simulink model "complex_iq_bw2048_out32_fixaxi_v12b"  (R2019a) (continued)
== system complex_iq_bw2048_out32_fixaxi_v12b/dec_fir2 == (continued)
      |         set_param_state(gcb, 'frac_width', 'on')
      |     else
      |         set_param_state(gcb, 'exp_width', 'off')
      |         set_param_state(gcb, 'frac_width', 'off')
      |     end
      | 
      | 
      | else
      |     enable_fixpt = 'on'
      |     set_param_state(gcb, 'float_type', 'off')
      |     set_param_state(gcb, 'exp_width', 'off')
      |     set_param_state(gcb, 'frac_width', 'off')
      | end
      mask TypeOptions float_type: single | custom
      mask Callback float_type:
      | f_type = get_param(gcb, 'float_type')
      | if strcmp(f_type,'custom')
      |     set_param_state(gcb, 'exp_width', 'on')
      |     set_param_state(gcb, 'frac_width', 'on')
      | else
      |     set_param_state(gcb, 'exp_width', 'off')
      |     set_param_state(gcb, 'frac_width', 'off')
      | end
  ri_to_c : casper_library_misc/ri_to_c
      settings: SourceProductName=CASPER DSP Blockset
  shift1 : xbsIndex_r4/Shift  shift_dir=Left  shift_bits=1  en=off  latency=1  precision=Full  arith_type="Signed  (2's comp)"  n_bits=16  bin_pt=14  quantization=Truncate  overflow=Wrap  dbl_ovrd=off  block_type=shift
  shift2 : xbsIndex_r4/Shift  shift_dir=Left  shift_bits=1  en=off  latency=1  precision=Full  arith_type="Signed  (2's comp)"  n_bits=16  bin_pt=14  quantization=Truncate  overflow=Wrap  dbl_ovrd=off  block_type=shift
  sync_out : Outport
  dout : Outport  Port=2
  wires:
  real1/1 -> fir_dbl_col1/1(real1)
  imag1/1 -> fir_dbl_col1/2(imag1)
  real2/1 -> fir_dbl_col1/3(real2)
  imag2/1 -> fir_dbl_col1/4(imag2)
  real3/1 -> fir_dbl_col1/5(real3)
  imag3/1 -> fir_dbl_col1/6(imag3)
  real4/1 -> fir_dbl_col1/7(real4)
  imag4/1 -> fir_dbl_col1/8(imag4)
  real5/1 -> fir_dbl_col1/9(real5)
  imag5/1 -> fir_dbl_col1/10(imag5)
  real6/1 -> fir_dbl_col1/11(real6)
  imag6/1 -> fir_dbl_col1/12(imag6)
  real7/1 -> fir_dbl_col1/13(real7)
  imag7/1 -> fir_dbl_col1/14(imag7)
  real8/1 -> fir_dbl_col1/15(real8)
  imag8/1 -> fir_dbl_col1/16(imag8)
  fir_dbl_col1/33(real_sum) -> real_sum/2(din1)
  fir_dbl_col1/34(imag_sum) -> imag_sum/2(din1)
  fir_dbl_col1/1(real_out1) -> fir_dbl_col2/1(real1)
  fir_dbl_col1/2(imag_out1) -> fir_dbl_col2/2(imag1)
  fir_dbl_col1/3(real_out2) -> fir_dbl_col2/3(real2)
  fir_dbl_col1/4(imag_out2) -> fir_dbl_col2/4(imag2)
  fir_dbl_col1/5(real_out3) -> fir_dbl_col2/5(real3)
  fir_dbl_col1/6(imag_out3) -> fir_dbl_col2/6(imag3)
  fir_dbl_col1/7(real_out4) -> fir_dbl_col2/7(real4)
  fir_dbl_col1/8(imag_out4) -> fir_dbl_col2/8(imag4)
  fir_dbl_col1/9(real_out5) -> fir_dbl_col2/9(real5)
  fir_dbl_col1/10(imag_out5) -> fir_dbl_col2/10(imag5)
  fir_dbl_col1/11(real_out6) -> fir_dbl_col2/11(real6)
  fir_dbl_col1/12(imag_out6) -> fir_dbl_col2/12(imag6)
  fir_dbl_col1/13(real_out7) -> fir_dbl_col2/13(real7)
  fir_dbl_col1/14(imag_out7) -> fir_dbl_col2/14(imag7)
  fir_dbl_col1/15(real_out8) -> fir_dbl_col2/15(real8)
  fir_dbl_col1/16(imag_out8) -> fir_dbl_col2/16(imag8)
  fir_dbl_col2/33(real_sum) -> real_sum/3(din2)
  fir_dbl_col2/34(imag_sum) -> imag_sum/3(din2)
  fir_dbl_col2/1(real_out1) -> fir_dbl_col3/1(real1)
  fir_dbl_col2/2(imag_out1) -> fir_dbl_col3/2(imag1)
  fir_dbl_col2/3(real_out2) -> fir_dbl_col3/3(real2)
  fir_dbl_col2/4(imag_out2) -> fir_dbl_col3/4(imag2)
  fir_dbl_col2/5(real_out3) -> fir_dbl_col3/5(real3)
  fir_dbl_col2/6(imag_out3) -> fir_dbl_col3/6(imag3)
  fir_dbl_col2/7(real_out4) -> fir_dbl_col3/7(real4)
  fir_dbl_col2/8(imag_out4) -> fir_dbl_col3/8(imag4)
  fir_dbl_col2/9(real_out5) -> fir_dbl_col3/9(real5)
  fir_dbl_col2/10(imag_out5) -> fir_dbl_col3/10(imag5)
  fir_dbl_col2/11(real_out6) -> fir_dbl_col3/11(real6)
  fir_dbl_col2/12(imag_out6) -> fir_dbl_col3/12(imag6)
  fir_dbl_col2/13(real_out7) -> fir_dbl_col3/13(real7)
  fir_dbl_col2/14(imag_out7) -> fir_dbl_col3/14(imag7)
  fir_dbl_col2/15(real_out8) -> fir_dbl_col3/15(real8)
  fir_dbl_col2/16(imag_out8) -> fir_dbl_col3/16(imag8)
  fir_dbl_col3/33(real_sum) -> real_sum/4(din3)
  fir_dbl_col3/34(imag_sum) -> imag_sum/4(din3)
  fir_dbl_col3/1(real_out1) -> fir_dbl_col4/1(real1)
  fir_dbl_col3/2(imag_out1) -> fir_dbl_col4/2(imag1)
  fir_dbl_col3/3(real_out2) -> fir_dbl_col4/3(real2)
  fir_dbl_col3/4(imag_out2) -> fir_dbl_col4/4(imag2)
  fir_dbl_col3/5(real_out3) -> fir_dbl_col4/5(real3)
  fir_dbl_col3/6(imag_out3) -> fir_dbl_col4/6(imag3)
  fir_dbl_col3/7(real_out4) -> fir_dbl_col4/7(real4)
  fir_dbl_col3/8(imag_out4) -> fir_dbl_col4/8(imag4)
  fir_dbl_col3/9(real_out5) -> fir_dbl_col4/9(real5)
  fir_dbl_col3/10(imag_out5) -> fir_dbl_col4/10(imag5)
  fir_dbl_col3/11(real_out6) -> fir_dbl_col4/11(real6)
  fir_dbl_col3/12(imag_out6) -> fir_dbl_col4/12(imag6)
  fir_dbl_col3/13(real_out7) -> fir_dbl_col4/13(real7)
  fir_dbl_col3/14(imag_out7) -> fir_dbl_col4/14(imag7)
  fir_dbl_col3/15(real_out8) -> fir_dbl_col4/15(real8)
  fir_dbl_col3/16(imag_out8) -> fir_dbl_col4/16(imag8)
  fir_dbl_col4/33(real_sum) -> real_sum/5(din4)
  fir_dbl_col4/34(imag_sum) -> imag_sum/5(din4)
  fir_dbl_col4/1(real_out1) -> fir_dbl_col5/1(real1)
  fir_dbl_col4/2(imag_out1) -> fir_dbl_col5/2(imag1)
  fir_dbl_col4/3(real_out2) -> fir_dbl_col5/3(real2)
  fir_dbl_col4/4(imag_out2) -> fir_dbl_col5/4(imag2)
  fir_dbl_col4/5(real_out3) -> fir_dbl_col5/5(real3)
  fir_dbl_col4/6(imag_out3) -> fir_dbl_col5/6(imag3)
  fir_dbl_col4/7(real_out4) -> fir_dbl_col5/7(real4)
  fir_dbl_col4/8(imag_out4) -> fir_dbl_col5/8(imag4)
  fir_dbl_col4/9(real_out5) -> fir_dbl_col5/9(real5)
  fir_dbl_col4/10(imag_out5) -> fir_dbl_col5/10(imag5)
  fir_dbl_col4/11(real_out6) -> fir_dbl_col5/11(real6)
  fir_dbl_col4/12(imag_out6) -> fir_dbl_col5/12(imag6)
  fir_dbl_col4/13(real_out7) -> fir_dbl_col5/13(real7)
  fir_dbl_col4/14(imag_out7) -> fir_dbl_col5/14(imag7)
  fir_dbl_col4/15(real_out8) -> fir_dbl_col5/15(real8)
  fir_dbl_col4/16(imag_out8) -> fir_dbl_col5/16(imag8)
  fir_dbl_col5/33(real_sum) -> real_sum/6(din5)
  fir_dbl_col5/34(imag_sum) -> imag_sum/6(din5)
  fir_dbl_col5/1(real_out1) -> fir_dbl_col6/1(real1)
  fir_dbl_col5/2(imag_out1) -> fir_dbl_col6/2(imag1)
  fir_dbl_col5/3(real_out2) -> fir_dbl_col6/3(real2)
  fir_dbl_col5/4(imag_out2) -> fir_dbl_col6/4(imag2)
  fir_dbl_col5/5(real_out3) -> fir_dbl_col6/5(real3)
  fir_dbl_col5/6(imag_out3) -> fir_dbl_col6/6(imag3)
  fir_dbl_col5/7(real_out4) -> fir_dbl_col6/7(real4)
  fir_dbl_col5/8(imag_out4) -> fir_dbl_col6/8(imag4)
  fir_dbl_col5/9(real_out5) -> fir_dbl_col6/9(real5)
  fir_dbl_col5/10(imag_out5) -> fir_dbl_col6/10(imag5)
  fir_dbl_col5/11(real_out6) -> fir_dbl_col6/11(real6)
  fir_dbl_col5/12(imag_out6) -> fir_dbl_col6/12(imag6)
  fir_dbl_col5/13(real_out7) -> fir_dbl_col6/13(real7)
  fir_dbl_col5/14(imag_out7) -> fir_dbl_col6/14(imag7)
  fir_dbl_col5/15(real_out8) -> fir_dbl_col6/15(real8)
  fir_dbl_col5/16(imag_out8) -> fir_dbl_col6/16(imag8)
  fir_dbl_col6/33(real_sum) -> real_sum/7(din6)
  fir_dbl_col6/34(imag_sum) -> imag_sum/7(din6)
  fir_dbl_col6/1(real_out1) -> fir_dbl_col7/1(real1)
  fir_dbl_col6/2(imag_out1) -> fir_dbl_col7/2(imag1)
  fir_dbl_col6/3(real_out2) -> fir_dbl_col7/3(real2)
  fir_dbl_col6/4(imag_out2) -> fir_dbl_col7/4(imag2)
  fir_dbl_col6/5(real_out3) -> fir_dbl_col7/5(real3)
  fir_dbl_col6/6(imag_out3) -> fir_dbl_col7/6(imag3)
  fir_dbl_col6/7(real_out4) -> fir_dbl_col7/7(real4)
  fir_dbl_col6/8(imag_out4) -> fir_dbl_col7/8(imag4)
  fir_dbl_col6/9(real_out5) -> fir_dbl_col7/9(real5)
  fir_dbl_col6/10(imag_out5) -> fir_dbl_col7/10(imag5)
  fir_dbl_col6/11(real_out6) -> fir_dbl_col7/11(real6)
  fir_dbl_col6/12(imag_out6) -> fir_dbl_col7/12(imag6)
  fir_dbl_col6/13(real_out7) -> fir_dbl_col7/13(real7)
  fir_dbl_col6/14(imag_out7) -> fir_dbl_col7/14(imag7)
  fir_dbl_col6/15(real_out8) -> fir_dbl_col7/15(real8)
  fir_dbl_col6/16(imag_out8) -> fir_dbl_col7/16(imag8)
  fir_dbl_col7/33(real_sum) -> real_sum/8(din7)
  fir_dbl_col7/34(imag_sum) -> imag_sum/8(din7)
  fir_dbl_col7/1(real_out1) -> fir_dbl_col8/1(real1)
  fir_dbl_col7/2(imag_out1) -> fir_dbl_col8/2(imag1)
  fir_dbl_col7/3(real_out2) -> fir_dbl_col8/3(real2)
  fir_dbl_col7/4(imag_out2) -> fir_dbl_col8/4(imag2)
  fir_dbl_col7/5(real_out3) -> fir_dbl_col8/5(real3)
  fir_dbl_col7/6(imag_out3) -> fir_dbl_col8/6(imag3)
  fir_dbl_col7/7(real_out4) -> fir_dbl_col8/7(real4)
  fir_dbl_col7/8(imag_out4) -> fir_dbl_col8/8(imag4)
  fir_dbl_col7/9(real_out5) -> fir_dbl_col8/9(real5)
  fir_dbl_col7/10(imag_out5) -> fir_dbl_col8/10(imag5)
  fir_dbl_col7/11(real_out6) -> fir_dbl_col8/11(real6)
  fir_dbl_col7/12(imag_out6) -> fir_dbl_col8/12(imag6)
  fir_dbl_col7/13(real_out7) -> fir_dbl_col8/13(real7)
  fir_dbl_col7/14(imag_out7) -> fir_dbl_col8/14(imag7)
  fir_dbl_col7/15(real_out8) -> fir_dbl_col8/15(real8)
  fir_dbl_col7/16(imag_out8) -> fir_dbl_col8/16(imag8)
  fir_dbl_col8/33(real_sum) -> real_sum/9(din8)
  fir_dbl_col8/34(imag_sum) -> imag_sum/9(din8)
  fir_dbl_col8/1(real_out1) -> fir_dbl_col9/1(real1)
  fir_dbl_col8/2(imag_out1) -> fir_dbl_col9/2(imag1)
  fir_dbl_col8/3(real_out2) -> fir_dbl_col9/3(real2)
  fir_dbl_col8/4(imag_out2) -> fir_dbl_col9/4(imag2)
  fir_dbl_col8/5(real_out3) -> fir_dbl_col9/5(real3)
  fir_dbl_col8/6(imag_out3) -> fir_dbl_col9/6(imag3)
  fir_dbl_col8/7(real_out4) -> fir_dbl_col9/7(real4)
  fir_dbl_col8/8(imag_out4) -> fir_dbl_col9/8(imag4)
  fir_dbl_col8/9(real_out5) -> fir_dbl_col9/9(real5)
  fir_dbl_col8/10(imag_out5) -> fir_dbl_col9/10(imag5)
  fir_dbl_col8/11(real_out6) -> fir_dbl_col9/11(real6)
  fir_dbl_col8/12(imag_out6) -> fir_dbl_col9/12(imag6)
  fir_dbl_col8/13(real_out7) -> fir_dbl_col9/13(real7)
  fir_dbl_col8/14(imag_out7) -> fir_dbl_col9/14(imag7)
  fir_dbl_col8/15(real_out8) -> fir_dbl_col9/15(real8)
  fir_dbl_col8/16(imag_out8) -> fir_dbl_col9/16(imag8)
  fir_dbl_col9/33(real_sum) -> real_sum/10(din9)
  fir_dbl_col9/34(imag_sum) -> imag_sum/10(din9)
  fir_dbl_col9/1(real_out1) -> fir_dbl_col10/1(real1)
  fir_dbl_col9/2(imag_out1) -> fir_dbl_col10/2(imag1)
  fir_dbl_col9/3(real_out2) -> fir_dbl_col10/3(real2)
  fir_dbl_col9/4(imag_out2) -> fir_dbl_col10/4(imag2)
  fir_dbl_col9/5(real_out3) -> fir_dbl_col10/5(real3)
  fir_dbl_col9/6(imag_out3) -> fir_dbl_col10/6(imag3)
  fir_dbl_col9/7(real_out4) -> fir_dbl_col10/7(real4)
  fir_dbl_col9/8(imag_out4) -> fir_dbl_col10/8(imag4)
  fir_dbl_col9/9(real_out5) -> fir_dbl_col10/9(real5)
  fir_dbl_col9/10(imag_out5) -> fir_dbl_col10/10(imag5)
  fir_dbl_col9/11(real_out6) -> fir_dbl_col10/11(real6)
  fir_dbl_col9/12(imag_out6) -> fir_dbl_col10/12(imag6)
  fir_dbl_col9/13(real_out7) -> fir_dbl_col10/13(real7)
  fir_dbl_col9/14(imag_out7) -> fir_dbl_col10/14(imag7)
  fir_dbl_col9/15(real_out8) -> fir_dbl_col10/15(real8)
  fir_dbl_col9/16(imag_out8) -> fir_dbl_col10/16(imag8)
  fir_dbl_col10/33(real_sum) -> real_sum/11(din10)
  fir_dbl_col10/34(imag_sum) -> imag_sum/11(din10)
  fir_dbl_col10/1(real_out1) -> fir_dbl_col11/1(real1)
  fir_dbl_col10/2(imag_out1) -> fir_dbl_col11/2(imag1)
  fir_dbl_col10/3(real_out2) -> fir_dbl_col11/3(real2)
  fir_dbl_col10/4(imag_out2) -> fir_dbl_col11/4(imag2)
  fir_dbl_col10/5(real_out3) -> fir_dbl_col11/5(real3)
  fir_dbl_col10/6(imag_out3) -> fir_dbl_col11/6(imag3)
  fir_dbl_col10/7(real_out4) -> fir_dbl_col11/7(real4)
  fir_dbl_col10/8(imag_out4) -> fir_dbl_col11/8(imag4)
  fir_dbl_col10/9(real_out5) -> fir_dbl_col11/9(real5)
  fir_dbl_col10/10(imag_out5) -> fir_dbl_col11/10(imag5)
  fir_dbl_col10/11(real_out6) -> fir_dbl_col11/11(real6)
  fir_dbl_col10/12(imag_out6) -> fir_dbl_col11/12(imag6)
  fir_dbl_col10/13(real_out7) -> fir_dbl_col11/13(real7)
  fir_dbl_col10/14(imag_out7) -> fir_dbl_col11/14(imag7)
  fir_dbl_col10/15(real_out8) -> fir_dbl_col11/15(real8)
  fir_dbl_col10/16(imag_out8) -> fir_dbl_col11/16(imag8)
  fir_dbl_col11/33(real_sum) -> real_sum/12(din11)
  fir_dbl_col11/34(imag_sum) -> imag_sum/12(din11)
  fir_dbl_col11/1(real_out1) -> fir_dbl_col12/1(real1)
  fir_dbl_col11/2(imag_out1) -> fir_dbl_col12/2(imag1)
  fir_dbl_col11/3(real_out2) -> fir_dbl_col12/3(real2)
  fir_dbl_col11/4(imag_out2) -> fir_dbl_col12/4(imag2)
  fir_dbl_col11/5(real_out3) -> fir_dbl_col12/5(real3)
  fir_dbl_col11/6(imag_out3) -> fir_dbl_col12/6(imag3)
  fir_dbl_col11/7(real_out4) -> fir_dbl_col12/7(real4)
  fir_dbl_col11/8(imag_out4) -> fir_dbl_col12/8(imag4)
  fir_dbl_col11/9(real_out5) -> fir_dbl_col12/9(real5)
  fir_dbl_col11/10(imag_out5) -> fir_dbl_col12/10(imag5)
  fir_dbl_col11/11(real_out6) -> fir_dbl_col12/11(real6)
  fir_dbl_col11/12(imag_out6) -> fir_dbl_col12/12(imag6)
  fir_dbl_col11/13(real_out7) -> fir_dbl_col12/13(real7)
  fir_dbl_col11/14(imag_out7) -> fir_dbl_col12/14(imag7)
  fir_dbl_col11/15(real_out8) -> fir_dbl_col12/15(real8)
  fir_dbl_col11/16(imag_out8) -> fir_dbl_col12/16(imag8)
  fir_dbl_col12/33(real_sum) -> real_sum/13(din12)
  fir_dbl_col12/34(imag_sum) -> imag_sum/13(din12)
  fir_dbl_col12/1(real_out1) -> fir_dbl_col13/1(real1)
  fir_dbl_col12/2(imag_out1) -> fir_dbl_col13/2(imag1)
  fir_dbl_col12/3(real_out2) -> fir_dbl_col13/3(real2)
  fir_dbl_col12/4(imag_out2) -> fir_dbl_col13/4(imag2)
  fir_dbl_col12/5(real_out3) -> fir_dbl_col13/5(real3)
  fir_dbl_col12/6(imag_out3) -> fir_dbl_col13/6(imag3)
  fir_dbl_col12/7(real_out4) -> fir_dbl_col13/7(real4)
  fir_dbl_col12/8(imag_out4) -> fir_dbl_col13/8(imag4)
  fir_dbl_col12/9(real_out5) -> fir_dbl_col13/9(real5)
  fir_dbl_col12/10(imag_out5) -> fir_dbl_col13/10(imag5)
  fir_dbl_col12/11(real_out6) -> fir_dbl_col13/11(real6)
  fir_dbl_col12/12(imag_out6) -> fir_dbl_col13/12(imag6)
  fir_dbl_col12/13(real_out7) -> fir_dbl_col13/13(real7)
  fir_dbl_col12/14(imag_out7) -> fir_dbl_col13/14(imag7)
  fir_dbl_col12/15(real_out8) -> fir_dbl_col13/15(real8)
  fir_dbl_col12/16(imag_out8) -> fir_dbl_col13/16(imag8)
  fir_dbl_col13/33(real_sum) -> real_sum/14(din13)
  fir_dbl_col13/34(imag_sum) -> imag_sum/14(din13)
  fir_dbl_col13/1(real_out1) -> fir_dbl_col14/1(real1)
  fir_dbl_col13/2(imag_out1) -> fir_dbl_col14/2(imag1)
  fir_dbl_col13/3(real_out2) -> fir_dbl_col14/3(real2)
  fir_dbl_col13/4(imag_out2) -> fir_dbl_col14/4(imag2)
  fir_dbl_col13/5(real_out3) -> fir_dbl_col14/5(real3)
  fir_dbl_col13/6(imag_out3) -> fir_dbl_col14/6(imag3)
  fir_dbl_col13/7(real_out4) -> fir_dbl_col14/7(real4)
  fir_dbl_col13/8(imag_out4) -> fir_dbl_col14/8(imag4)
  fir_dbl_col13/9(real_out5) -> fir_dbl_col14/9(real5)
  fir_dbl_col13/10(imag_out5) -> fir_dbl_col14/10(imag5)
  fir_dbl_col13/11(real_out6) -> fir_dbl_col14/11(real6)
  fir_dbl_col13/12(imag_out6) -> fir_dbl_col14/12(imag6)
  fir_dbl_col13/13(real_out7) -> fir_dbl_col14/13(real7)
  fir_dbl_col13/14(imag_out7) -> fir_dbl_col14/14(imag7)
  fir_dbl_col13/15(real_out8) -> fir_dbl_col14/15(real8)
  fir_dbl_col13/16(imag_out8) -> fir_dbl_col14/16(imag8)
  fir_dbl_col14/33(real_sum) -> real_sum/15(din14)
  fir_dbl_col14/34(imag_sum) -> imag_sum/15(din14)
  fir_dbl_col14/1(real_out1) -> fir_dbl_col15/1(real1)
  fir_dbl_col14/2(imag_out1) -> fir_dbl_col15/2(imag1)
  fir_dbl_col14/3(real_out2) -> fir_dbl_col15/3(real2)
  fir_dbl_col14/4(imag_out2) -> fir_dbl_col15/4(imag2)
  fir_dbl_col14/5(real_out3) -> fir_dbl_col15/5(real3)
  fir_dbl_col14/6(imag_out3) -> fir_dbl_col15/6(imag3)
  fir_dbl_col14/7(real_out4) -> fir_dbl_col15/7(real4)
  fir_dbl_col14/8(imag_out4) -> fir_dbl_col15/8(imag4)
  fir_dbl_col14/9(real_out5) -> fir_dbl_col15/9(real5)
  fir_dbl_col14/10(imag_out5) -> fir_dbl_col15/10(imag5)
  fir_dbl_col14/11(real_out6) -> fir_dbl_col15/11(real6)
  fir_dbl_col14/12(imag_out6) -> fir_dbl_col15/12(imag6)
  fir_dbl_col14/13(real_out7) -> fir_dbl_col15/13(real7)
  fir_dbl_col14/14(imag_out7) -> fir_dbl_col15/14(imag7)
  fir_dbl_col14/15(real_out8) -> fir_dbl_col15/15(real8)
  fir_dbl_col14/16(imag_out8) -> fir_dbl_col15/16(imag8)
  fir_dbl_col15/33(real_sum) -> real_sum/16(din15)
  fir_dbl_col15/34(imag_sum) -> imag_sum/16(din15)
  fir_dbl_col15/1(real_out1) -> fir_dbl_col16/1(real1)
  fir_dbl_col15/2(imag_out1) -> fir_dbl_col16/2(imag1)
  fir_dbl_col15/3(real_out2) -> fir_dbl_col16/3(real2)
  fir_dbl_col15/4(imag_out2) -> fir_dbl_col16/4(imag2)
  fir_dbl_col15/5(real_out3) -> fir_dbl_col16/5(real3)
  fir_dbl_col15/6(imag_out3) -> fir_dbl_col16/6(imag3)
  fir_dbl_col15/7(real_out4) -> fir_dbl_col16/7(real4)
  fir_dbl_col15/8(imag_out4) -> fir_dbl_col16/8(imag4)
  fir_dbl_col15/9(real_out5) -> fir_dbl_col16/9(real5)
  fir_dbl_col15/10(imag_out5) -> fir_dbl_col16/10(imag5)
  fir_dbl_col15/11(real_out6) -> fir_dbl_col16/11(real6)
  fir_dbl_col15/12(imag_out6) -> fir_dbl_col16/12(imag6)
  fir_dbl_col15/13(real_out7) -> fir_dbl_col16/13(real7)
  fir_dbl_col15/14(imag_out7) -> fir_dbl_col16/14(imag7)
  fir_dbl_col15/15(real_out8) -> fir_dbl_col16/15(real8)
  fir_dbl_col15/16(imag_out8) -> fir_dbl_col16/16(imag8)
  fir_dbl_col16/33(real_sum) -> real_sum/17(din16)
  fir_dbl_col16/34(imag_sum) -> imag_sum/17(din16)
  fir_dbl_col16/1(real_out1) -> fir_dbl_col17/1(real1)
  fir_dbl_col16/2(imag_out1) -> fir_dbl_col17/2(imag1)
  fir_dbl_col16/3(real_out2) -> fir_dbl_col17/3(real2)
  fir_dbl_col16/4(imag_out2) -> fir_dbl_col17/4(imag2)
  fir_dbl_col16/5(real_out3) -> fir_dbl_col17/5(real3)
  fir_dbl_col16/6(imag_out3) -> fir_dbl_col17/6(imag3)
  fir_dbl_col16/7(real_out4) -> fir_dbl_col17/7(real4)
  fir_dbl_col16/8(imag_out4) -> fir_dbl_col17/8(imag4)
  fir_dbl_col16/9(real_out5) -> fir_dbl_col17/9(real5)
  fir_dbl_col16/10(imag_out5) -> fir_dbl_col17/10(imag5)
  fir_dbl_col16/11(real_out6) -> fir_dbl_col17/11(real6)
  fir_dbl_col16/12(imag_out6) -> fir_dbl_col17/12(imag6)
  fir_dbl_col16/13(real_out7) -> fir_dbl_col17/13(real7)
  fir_dbl_col16/14(imag_out7) -> fir_dbl_col17/14(imag7)
  fir_dbl_col16/15(real_out8) -> fir_dbl_col17/15(real8)
  fir_dbl_col16/16(imag_out8) -> fir_dbl_col17/16(imag8)
  fir_dbl_col17/33(real_sum) -> real_sum/18(din17)
  fir_dbl_col17/34(imag_sum) -> imag_sum/18(din17)
  fir_dbl_col17/1(real_out1) -> fir_dbl_col18/1(real1)
  fir_dbl_col17/2(imag_out1) -> fir_dbl_col18/2(imag1)
  fir_dbl_col17/3(real_out2) -> fir_dbl_col18/3(real2)
  fir_dbl_col17/4(imag_out2) -> fir_dbl_col18/4(imag2)
  fir_dbl_col17/5(real_out3) -> fir_dbl_col18/5(real3)
  fir_dbl_col17/6(imag_out3) -> fir_dbl_col18/6(imag3)
  fir_dbl_col17/7(real_out4) -> fir_dbl_col18/7(real4)
  fir_dbl_col17/8(imag_out4) -> fir_dbl_col18/8(imag4)
  fir_dbl_col17/9(real_out5) -> fir_dbl_col18/9(real5)
  fir_dbl_col17/10(imag_out5) -> fir_dbl_col18/10(imag5)
  fir_dbl_col17/11(real_out6) -> fir_dbl_col18/11(real6)
  fir_dbl_col17/12(imag_out6) -> fir_dbl_col18/12(imag6)
  fir_dbl_col17/13(real_out7) -> fir_dbl_col18/13(real7)
  fir_dbl_col17/14(imag_out7) -> fir_dbl_col18/14(imag7)
  fir_dbl_col17/15(real_out8) -> fir_dbl_col18/15(real8)
  fir_dbl_col17/16(imag_out8) -> fir_dbl_col18/16(imag8)
  fir_dbl_col18/33(real_sum) -> real_sum/19(din18)
  fir_dbl_col18/34(imag_sum) -> imag_sum/19(din18)
  fir_dbl_col18/1(real_out1) -> fir_dbl_col19/1(real1)
  fir_dbl_col18/2(imag_out1) -> fir_dbl_col19/2(imag1)
  fir_dbl_col18/3(real_out2) -> fir_dbl_col19/3(real2)
  fir_dbl_col18/4(imag_out2) -> fir_dbl_col19/4(imag2)
  fir_dbl_col18/5(real_out3) -> fir_dbl_col19/5(real3)
  fir_dbl_col18/6(imag_out3) -> fir_dbl_col19/6(imag3)
  fir_dbl_col18/7(real_out4) -> fir_dbl_col19/7(real4)
  fir_dbl_col18/8(imag_out4) -> fir_dbl_col19/8(imag4)
  fir_dbl_col18/9(real_out5) -> fir_dbl_col19/9(real5)
  fir_dbl_col18/10(imag_out5) -> fir_dbl_col19/10(imag5)
  fir_dbl_col18/11(real_out6) -> fir_dbl_col19/11(real6)
  fir_dbl_col18/12(imag_out6) -> fir_dbl_col19/12(imag6)
  fir_dbl_col18/13(real_out7) -> fir_dbl_col19/13(real7)
  fir_dbl_col18/14(imag_out7) -> fir_dbl_col19/14(imag7)
  fir_dbl_col18/15(real_out8) -> fir_dbl_col19/15(real8)
  fir_dbl_col18/16(imag_out8) -> fir_dbl_col19/16(imag8)
  fir_dbl_col19/33(real_sum) -> real_sum/20(din19)
  fir_dbl_col19/34(imag_sum) -> imag_sum/20(din19)
  fir_dbl_col19/1(real_out1) -> fir_dbl_col20/1(real1)
  fir_dbl_col19/2(imag_out1) -> fir_dbl_col20/2(imag1)
  fir_dbl_col19/3(real_out2) -> fir_dbl_col20/3(real2)
  fir_dbl_col19/4(imag_out2) -> fir_dbl_col20/4(imag2)
  fir_dbl_col19/5(real_out3) -> fir_dbl_col20/5(real3)
  fir_dbl_col19/6(imag_out3) -> fir_dbl_col20/6(imag3)
  fir_dbl_col19/7(real_out4) -> fir_dbl_col20/7(real4)
  fir_dbl_col19/8(imag_out4) -> fir_dbl_col20/8(imag4)
  fir_dbl_col19/9(real_out5) -> fir_dbl_col20/9(real5)
  fir_dbl_col19/10(imag_out5) -> fir_dbl_col20/10(imag5)
  fir_dbl_col19/11(real_out6) -> fir_dbl_col20/11(real6)
  fir_dbl_col19/12(imag_out6) -> fir_dbl_col20/12(imag6)
  fir_dbl_col19/13(real_out7) -> fir_dbl_col20/13(real7)
  fir_dbl_col19/14(imag_out7) -> fir_dbl_col20/14(imag7)
  fir_dbl_col19/15(real_out8) -> fir_dbl_col20/15(real8)
  fir_dbl_col19/16(imag_out8) -> fir_dbl_col20/16(imag8)
  fir_dbl_col20/33(real_sum) -> real_sum/21(din20)
  fir_dbl_col20/34(imag_sum) -> imag_sum/21(din20)
  fir_dbl_col20/1(real_out1) -> fir_dbl_col21/1(real1)
  fir_dbl_col20/2(imag_out1) -> fir_dbl_col21/2(imag1)
  fir_dbl_col20/3(real_out2) -> fir_dbl_col21/3(real2)
  fir_dbl_col20/4(imag_out2) -> fir_dbl_col21/4(imag2)
  fir_dbl_col20/5(real_out3) -> fir_dbl_col21/5(real3)
  fir_dbl_col20/6(imag_out3) -> fir_dbl_col21/6(imag3)
  fir_dbl_col20/7(real_out4) -> fir_dbl_col21/7(real4)
  fir_dbl_col20/8(imag_out4) -> fir_dbl_col21/8(imag4)
  fir_dbl_col20/9(real_out5) -> fir_dbl_col21/9(real5)
  fir_dbl_col20/10(imag_out5) -> fir_dbl_col21/10(imag5)
  fir_dbl_col20/11(real_out6) -> fir_dbl_col21/11(real6)
  fir_dbl_col20/12(imag_out6) -> fir_dbl_col21/12(imag6)
  fir_dbl_col20/13(real_out7) -> fir_dbl_col21/13(real7)
  fir_dbl_col20/14(imag_out7) -> fir_dbl_col21/14(imag7)
  fir_dbl_col20/15(real_out8) -> fir_dbl_col21/15(real8)
  fir_dbl_col20/16(imag_out8) -> fir_dbl_col21/16(imag8)
  fir_dbl_col21/33(real_sum) -> real_sum/22(din21)
  fir_dbl_col21/34(imag_sum) -> imag_sum/22(din21)
  fir_dbl_col21/1(real_out1) -> fir_dbl_col22/1(real1)
  fir_dbl_col21/2(imag_out1) -> fir_dbl_col22/2(imag1)
  fir_dbl_col21/3(real_out2) -> fir_dbl_col22/3(real2)
  fir_dbl_col21/4(imag_out2) -> fir_dbl_col22/4(imag2)
  fir_dbl_col21/5(real_out3) -> fir_dbl_col22/5(real3)
  fir_dbl_col21/6(imag_out3) -> fir_dbl_col22/6(imag3)
  fir_dbl_col21/7(real_out4) -> fir_dbl_col22/7(real4)
  fir_dbl_col21/8(imag_out4) -> fir_dbl_col22/8(imag4)
  fir_dbl_col21/9(real_out5) -> fir_dbl_col22/9(real5)
  fir_dbl_col21/10(imag_out5) -> fir_dbl_col22/10(imag5)
  fir_dbl_col21/11(real_out6) -> fir_dbl_col22/11(real6)
  fir_dbl_col21/12(imag_out6) -> fir_dbl_col22/12(imag6)
  fir_dbl_col21/13(real_out7) -> fir_dbl_col22/13(real7)
  fir_dbl_col21/14(imag_out7) -> fir_dbl_col22/14(imag7)
  fir_dbl_col21/15(real_out8) -> fir_dbl_col22/15(real8)
  fir_dbl_col21/16(imag_out8) -> fir_dbl_col22/16(imag8)
  fir_dbl_col22/33(real_sum) -> real_sum/23(din22)
  fir_dbl_col22/34(imag_sum) -> imag_sum/23(din22)
  fir_dbl_col22/1(real_out1) -> fir_dbl_col23/1(real1)
  fir_dbl_col22/2(imag_out1) -> fir_dbl_col23/2(imag1)
  fir_dbl_col22/3(real_out2) -> fir_dbl_col23/3(real2)
  fir_dbl_col22/4(imag_out2) -> fir_dbl_col23/4(imag2)
  fir_dbl_col22/5(real_out3) -> fir_dbl_col23/5(real3)
  fir_dbl_col22/6(imag_out3) -> fir_dbl_col23/6(imag3)
  fir_dbl_col22/7(real_out4) -> fir_dbl_col23/7(real4)
  fir_dbl_col22/8(imag_out4) -> fir_dbl_col23/8(imag4)
  fir_dbl_col22/9(real_out5) -> fir_dbl_col23/9(real5)
  fir_dbl_col22/10(imag_out5) -> fir_dbl_col23/10(imag5)
  fir_dbl_col22/11(real_out6) -> fir_dbl_col23/11(real6)
  fir_dbl_col22/12(imag_out6) -> fir_dbl_col23/12(imag6)
  fir_dbl_col22/13(real_out7) -> fir_dbl_col23/13(real7)
  fir_dbl_col22/14(imag_out7) -> fir_dbl_col23/14(imag7)
  fir_dbl_col22/15(real_out8) -> fir_dbl_col23/15(real8)
  fir_dbl_col22/16(imag_out8) -> fir_dbl_col23/16(imag8)
  fir_dbl_col23/33(real_sum) -> real_sum/24(din23)
  fir_dbl_col23/34(imag_sum) -> imag_sum/24(din23)
  fir_dbl_col23/1(real_out1) -> fir_dbl_col24/1(real1)
  fir_dbl_col23/2(imag_out1) -> fir_dbl_col24/2(imag1)
  fir_dbl_col23/3(real_out2) -> fir_dbl_col24/3(real2)
  fir_dbl_col23/4(imag_out2) -> fir_dbl_col24/4(imag2)
  fir_dbl_col23/5(real_out3) -> fir_dbl_col24/5(real3)
  fir_dbl_col23/6(imag_out3) -> fir_dbl_col24/6(imag3)
  fir_dbl_col23/7(real_out4) -> fir_dbl_col24/7(real4)
  fir_dbl_col23/8(imag_out4) -> fir_dbl_col24/8(imag4)
  fir_dbl_col23/9(real_out5) -> fir_dbl_col24/9(real5)
  fir_dbl_col23/10(imag_out5) -> fir_dbl_col24/10(imag5)
  fir_dbl_col23/11(real_out6) -> fir_dbl_col24/11(real6)
  fir_dbl_col23/12(imag_out6) -> fir_dbl_col24/12(imag6)
  fir_dbl_col23/13(real_out7) -> fir_dbl_col24/13(real7)
  fir_dbl_col23/14(imag_out7) -> fir_dbl_col24/14(imag7)
  fir_dbl_col23/15(real_out8) -> fir_dbl_col24/15(real8)
  fir_dbl_col23/16(imag_out8) -> fir_dbl_col24/16(imag8)
  fir_dbl_col24/33(real_sum) -> real_sum/25(din24)
  fir_dbl_col24/34(imag_sum) -> imag_sum/25(din24)
  fir_dbl_col24/1(real_out1) -> fir_dbl_col25/1(real1)
  fir_dbl_col24/2(imag_out1) -> fir_dbl_col25/2(imag1)
  fir_dbl_col24/3(real_out2) -> fir_dbl_col25/3(real2)
  fir_dbl_col24/4(imag_out2) -> fir_dbl_col25/4(imag2)
  fir_dbl_col24/5(real_out3) -> fir_dbl_col25/5(real3)
  fir_dbl_col24/6(imag_out3) -> fir_dbl_col25/6(imag3)
  fir_dbl_col24/7(real_out4) -> fir_dbl_col25/7(real4)
  fir_dbl_col24/8(imag_out4) -> fir_dbl_col25/8(imag4)
  fir_dbl_col24/9(real_out5) -> fir_dbl_col25/9(real5)
  fir_dbl_col24/10(imag_out5) -> fir_dbl_col25/10(imag5)
  fir_dbl_col24/11(real_out6) -> fir_dbl_col25/11(real6)
  fir_dbl_col24/12(imag_out6) -> fir_dbl_col25/12(imag6)
  fir_dbl_col24/13(real_out7) -> fir_dbl_col25/13(real7)
  fir_dbl_col24/14(imag_out7) -> fir_dbl_col25/14(imag7)
  fir_dbl_col24/15(real_out8) -> fir_dbl_col25/15(real8)
  fir_dbl_col24/16(imag_out8) -> fir_dbl_col25/16(imag8)
  fir_dbl_col25/33(real_sum) -> real_sum/26(din25)
  fir_dbl_col25/34(imag_sum) -> imag_sum/26(din25)
  fir_dbl_col25/1(real_out1) -> fir_dbl_col26/1(real1)
  fir_dbl_col25/2(imag_out1) -> fir_dbl_col26/2(imag1)
  fir_dbl_col25/3(real_out2) -> fir_dbl_col26/3(real2)
  fir_dbl_col25/4(imag_out2) -> fir_dbl_col26/4(imag2)
  fir_dbl_col25/5(real_out3) -> fir_dbl_col26/5(real3)
  fir_dbl_col25/6(imag_out3) -> fir_dbl_col26/6(imag3)
  fir_dbl_col25/7(real_out4) -> fir_dbl_col26/7(real4)
  fir_dbl_col25/8(imag_out4) -> fir_dbl_col26/8(imag4)
  fir_dbl_col25/9(real_out5) -> fir_dbl_col26/9(real5)
  fir_dbl_col25/10(imag_out5) -> fir_dbl_col26/10(imag5)
  fir_dbl_col25/11(real_out6) -> fir_dbl_col26/11(real6)
  fir_dbl_col25/12(imag_out6) -> fir_dbl_col26/12(imag6)
  fir_dbl_col25/13(real_out7) -> fir_dbl_col26/13(real7)
  fir_dbl_col25/14(imag_out7) -> fir_dbl_col26/14(imag7)
  fir_dbl_col25/15(real_out8) -> fir_dbl_col26/15(real8)
  fir_dbl_col25/16(imag_out8) -> fir_dbl_col26/16(imag8)
  fir_dbl_col26/33(real_sum) -> real_sum/27(din26)
  fir_dbl_col26/34(imag_sum) -> imag_sum/27(din26)
  fir_dbl_col26/1(real_out1) -> fir_dbl_col27/1(real1)
  fir_dbl_col26/2(imag_out1) -> fir_dbl_col27/2(imag1)
  fir_dbl_col26/3(real_out2) -> fir_dbl_col27/3(real2)
  fir_dbl_col26/4(imag_out2) -> fir_dbl_col27/4(imag2)
  fir_dbl_col26/5(real_out3) -> fir_dbl_col27/5(real3)
  fir_dbl_col26/6(imag_out3) -> fir_dbl_col27/6(imag3)
  fir_dbl_col26/7(real_out4) -> fir_dbl_col27/7(real4)
  fir_dbl_col26/8(imag_out4) -> fir_dbl_col27/8(imag4)
  fir_dbl_col26/9(real_out5) -> fir_dbl_col27/9(real5)
  fir_dbl_col26/10(imag_out5) -> fir_dbl_col27/10(imag5)
  fir_dbl_col26/11(real_out6) -> fir_dbl_col27/11(real6)
  fir_dbl_col26/12(imag_out6) -> fir_dbl_col27/12(imag6)
  fir_dbl_col26/13(real_out7) -> fir_dbl_col27/13(real7)
  fir_dbl_col26/14(imag_out7) -> fir_dbl_col27/14(imag7)
  fir_dbl_col26/15(real_out8) -> fir_dbl_col27/15(real8)
  fir_dbl_col26/16(imag_out8) -> fir_dbl_col27/16(imag8)
  fir_dbl_col27/33(real_sum) -> real_sum/28(din27)
  fir_dbl_col27/34(imag_sum) -> imag_sum/28(din27)
  fir_dbl_col27/1(real_out1) -> fir_dbl_col28/1(real1)
  fir_dbl_col27/2(imag_out1) -> fir_dbl_col28/2(imag1)
  fir_dbl_col27/3(real_out2) -> fir_dbl_col28/3(real2)
  fir_dbl_col27/4(imag_out2) -> fir_dbl_col28/4(imag2)
  fir_dbl_col27/5(real_out3) -> fir_dbl_col28/5(real3)
  fir_dbl_col27/6(imag_out3) -> fir_dbl_col28/6(imag3)
  fir_dbl_col27/7(real_out4) -> fir_dbl_col28/7(real4)
  fir_dbl_col27/8(imag_out4) -> fir_dbl_col28/8(imag4)
  fir_dbl_col27/9(real_out5) -> fir_dbl_col28/9(real5)
  fir_dbl_col27/10(imag_out5) -> fir_dbl_col28/10(imag5)
  fir_dbl_col27/11(real_out6) -> fir_dbl_col28/11(real6)
  fir_dbl_col27/12(imag_out6) -> fir_dbl_col28/12(imag6)
  fir_dbl_col27/13(real_out7) -> fir_dbl_col28/13(real7)
  fir_dbl_col27/14(imag_out7) -> fir_dbl_col28/14(imag7)
  fir_dbl_col27/15(real_out8) -> fir_dbl_col28/15(real8)
  fir_dbl_col27/16(imag_out8) -> fir_dbl_col28/16(imag8)
  fir_dbl_col28/33(real_sum) -> real_sum/29(din28)
  fir_dbl_col28/34(imag_sum) -> imag_sum/29(din28)
  fir_dbl_col28/1(real_out1) -> fir_dbl_col29/1(real1)
  fir_dbl_col28/2(imag_out1) -> fir_dbl_col29/2(imag1)
  fir_dbl_col28/3(real_out2) -> fir_dbl_col29/3(real2)
  fir_dbl_col28/4(imag_out2) -> fir_dbl_col29/4(imag2)
  fir_dbl_col28/5(real_out3) -> fir_dbl_col29/5(real3)
  fir_dbl_col28/6(imag_out3) -> fir_dbl_col29/6(imag3)
  fir_dbl_col28/7(real_out4) -> fir_dbl_col29/7(real4)
  fir_dbl_col28/8(imag_out4) -> fir_dbl_col29/8(imag4)
  fir_dbl_col28/9(real_out5) -> fir_dbl_col29/9(real5)
  fir_dbl_col28/10(imag_out5) -> fir_dbl_col29/10(imag5)
  fir_dbl_col28/11(real_out6) -> fir_dbl_col29/11(real6)
  fir_dbl_col28/12(imag_out6) -> fir_dbl_col29/12(imag6)
  fir_dbl_col28/13(real_out7) -> fir_dbl_col29/13(real7)
  fir_dbl_col28/14(imag_out7) -> fir_dbl_col29/14(imag7)
  fir_dbl_col28/15(real_out8) -> fir_dbl_col29/15(real8)
  fir_dbl_col28/16(imag_out8) -> fir_dbl_col29/16(imag8)
  fir_dbl_col29/33(real_sum) -> real_sum/30(din29)
  fir_dbl_col29/34(imag_sum) -> imag_sum/30(din29)
  fir_dbl_col29/1(real_out1) -> fir_dbl_col30/1(real1)
  fir_dbl_col29/2(imag_out1) -> fir_dbl_col30/2(imag1)
  fir_dbl_col29/3(real_out2) -> fir_dbl_col30/3(real2)
  fir_dbl_col29/4(imag_out2) -> fir_dbl_col30/4(imag2)
  fir_dbl_col29/5(real_out3) -> fir_dbl_col30/5(real3)
  fir_dbl_col29/6(imag_out3) -> fir_dbl_col30/6(imag3)
  fir_dbl_col29/7(real_out4) -> fir_dbl_col30/7(real4)
  fir_dbl_col29/8(imag_out4) -> fir_dbl_col30/8(imag4)
  fir_dbl_col29/9(real_out5) -> fir_dbl_col30/9(real5)
  fir_dbl_col29/10(imag_out5) -> fir_dbl_col30/10(imag5)
  fir_dbl_col29/11(real_out6) -> fir_dbl_col30/11(real6)
  fir_dbl_col29/12(imag_out6) -> fir_dbl_col30/12(imag6)
  fir_dbl_col29/13(real_out7) -> fir_dbl_col30/13(real7)
  fir_dbl_col29/14(imag_out7) -> fir_dbl_col30/14(imag7)
  fir_dbl_col29/15(real_out8) -> fir_dbl_col30/15(real8)
  fir_dbl_col29/16(imag_out8) -> fir_dbl_col30/16(imag8)
  fir_dbl_col30/33(real_sum) -> real_sum/31(din30)
  fir_dbl_col30/34(imag_sum) -> imag_sum/31(din30)
  fir_dbl_col30/1(real_out1) -> fir_dbl_col31/1(real1)
  fir_dbl_col30/2(imag_out1) -> fir_dbl_col31/2(imag1)
  fir_dbl_col30/3(real_out2) -> fir_dbl_col31/3(real2)
  fir_dbl_col30/4(imag_out2) -> fir_dbl_col31/4(imag2)
  fir_dbl_col30/5(real_out3) -> fir_dbl_col31/5(real3)
  fir_dbl_col30/6(imag_out3) -> fir_dbl_col31/6(imag3)
  fir_dbl_col30/7(real_out4) -> fir_dbl_col31/7(real4)
  fir_dbl_col30/8(imag_out4) -> fir_dbl_col31/8(imag4)
  fir_dbl_col30/9(real_out5) -> fir_dbl_col31/9(real5)
  fir_dbl_col30/10(imag_out5) -> fir_dbl_col31/10(imag5)
  fir_dbl_col30/11(real_out6) -> fir_dbl_col31/11(real6)
  fir_dbl_col30/12(imag_out6) -> fir_dbl_col31/12(imag6)
  fir_dbl_col30/13(real_out7) -> fir_dbl_col31/13(real7)
  fir_dbl_col30/14(imag_out7) -> fir_dbl_col31/14(imag7)
  fir_dbl_col30/15(real_out8) -> fir_dbl_col31/15(real8)
  fir_dbl_col30/16(imag_out8) -> fir_dbl_col31/16(imag8)
  fir_dbl_col31/33(real_sum) -> real_sum/32(din31)
  fir_dbl_col31/34(imag_sum) -> imag_sum/32(din31)
  fir_dbl_col31/1(real_out1) -> fir_dbl_col32/1(real1)
  fir_dbl_col31/2(imag_out1) -> fir_dbl_col32/2(imag1)
  fir_dbl_col31/3(real_out2) -> fir_dbl_col32/3(real2)
  fir_dbl_col31/4(imag_out2) -> fir_dbl_col32/4(imag2)
  fir_dbl_col31/5(real_out3) -> fir_dbl_col32/5(real3)
  fir_dbl_col31/6(imag_out3) -> fir_dbl_col32/6(imag3)
  fir_dbl_col31/7(real_out4) -> fir_dbl_col32/7(real4)
  fir_dbl_col31/8(imag_out4) -> fir_dbl_col32/8(imag4)
  fir_dbl_col31/9(real_out5) -> fir_dbl_col32/9(real5)
  fir_dbl_col31/10(imag_out5) -> fir_dbl_col32/10(imag5)
  fir_dbl_col31/11(real_out6) -> fir_dbl_col32/11(real6)
  fir_dbl_col31/12(imag_out6) -> fir_dbl_col32/12(imag6)
  fir_dbl_col31/13(real_out7) -> fir_dbl_col32/13(real7)
  fir_dbl_col31/14(imag_out7) -> fir_dbl_col32/14(imag7)
  fir_dbl_col31/15(real_out8) -> fir_dbl_col32/15(real8)
  fir_dbl_col31/16(imag_out8) -> fir_dbl_col32/16(imag8)
  fir_dbl_col32/33(real_sum) -> real_sum/33(din32)
  fir_dbl_col32/34(imag_sum) -> imag_sum/33(din32)
  real_sum/2(dout) -> shift1/1
  imag_sum/2(dout) -> shift2/1
  shift1/1 -> convert1/1
  shift2/1 -> convert2/1
  convert1/1 -> ri_to_c/1
  convert2/1 -> ri_to_c/2
  ri_to_c/1 -> dout/1
  sync_in/1 -> delay/1
  delay/1 -> real_sum/1(sync), imag_sum/1(sync)
  real_sum/1(sync_out) -> sync_out/1
  fir_dbl_col2/17(real_back_out1) -> fir_dbl_col1/17(real_back1)
  fir_dbl_col2/18(imag_back_out1) -> fir_dbl_col1/18(imag_back1)
  fir_dbl_col2/19(real_back_out2) -> fir_dbl_col1/19(real_back2)
  fir_dbl_col2/20(imag_back_out2) -> fir_dbl_col1/20(imag_back2)
  fir_dbl_col2/21(real_back_out3) -> fir_dbl_col1/21(real_back3)
  fir_dbl_col2/22(imag_back_out3) -> fir_dbl_col1/22(imag_back3)
  fir_dbl_col2/23(real_back_out4) -> fir_dbl_col1/23(real_back4)
  fir_dbl_col2/24(imag_back_out4) -> fir_dbl_col1/24(imag_back4)